simulink model "CCMSysServ"  (R2022b) (continued)
== system CCMSysServ == (continued)
      | TINTED TAILGATE WINDOW
      | <to>All</to>
      | <typ>Internal</typ>
      | <ReqID>NULL</ReqID>
      | <InitValue>0</InitValue>
  CarCfg_CarCfg189 : Inport  Description=|  Port=186  OutDataTypeStr=Nr2  PortDimensions=1
      | REAR SEAT VENTILATION
      | <to>All</to>
      | <typ>Internal</typ>
      | <ReqID>NULL</ReqID>
      | <InitValue>0</InitValue>
  CarCfg_CarCfg19 : Inport  Description=|  Port=187  OutDataTypeStr=Nr2  PortDimensions=1
      | TYRE PRESSURE MONITORING SYSTEM
      | <to>All</to>
      | <typ>Internal</typ>
      | <ReqID>NULL</ReqID>
      | <InitValue>0</InitValue>
  CarCfg_CarCfg190 : Inport  Description=|  Port=188  OutDataTypeStr=Nr2  PortDimensions=1
      | GLOBAL NAVIGATION SAT. SYSTEM RECEIVER
      | <to>All</to>
      | <typ>Internal</typ>
      | <ReqID>NULL</ReqID>
      | <InitValue>0</InitValue>
  CarCfg_CarCfg191 : Inport  Description=|  Port=189  OutDataTypeStr=Nr2  PortDimensions=1
      | TELEMATIC MODULE
      | <to>All</to>
      | <typ>Internal</typ>
      | <ReqID>NULL</ReqID>
      | <InitValue>0</InitValue>
  CarCfg_CarCfg192 : Inport  Description=|  Port=190  OutDataTypeStr=Nr2  PortDimensions=1
      | IHU LEVEL
      | <to>All</to>
      | <typ>Internal</typ>
      | <ReqID>NULL</ReqID>
      | <InitValue>0</InitValue>
  CarCfg_CarCfg193 : Inport  Description=|  Port=191  OutDataTypeStr=Nr2  PortDimensions=1
      | DIGITAL RADIO RECEIVER
      | <to>All</to>
      | <typ>Internal</typ>
      | <ReqID>NULL</ReqID>
      | <InitValue>0</InitValue>
  CarCfg_CarCfg194 : Inport  Description=|  Port=192  OutDataTypeStr=Nr2  PortDimensions=1
      | REAR SEAT ENTERTAINMENT
      | <to>All</to>
      | <typ>Internal</typ>
      | <ReqID>NULL</ReqID>
      | <InitValue>0</InitValue>
  CarCfg_CarCfg195 : Inport  Description=|  Port=193  OutDataTypeStr=Nr2  PortDimensions=1
      | REGION IF DVD
      | <to>All</to>
      | <typ>Internal</typ>
      | <ReqID>NULL</ReqID>
      | <InitValue>0</InitValue>
  CarCfg_CarCfg196 : Inport  Description=|  Port=194  OutDataTypeStr=Nr2  PortDimensions=1
      | Wi-Fi FREQUENCY BAND
      | <to>All</to>
      | <typ>Internal</typ>
      | <ReqID>NULL</ReqID>
      | <InitValue>0</InitValue>
  CarCfg_CarCfg197 : Inport  Description=|  Port=195  OutDataTypeStr=Nr2  PortDimensions=1
      | SYSTEM LANGUAGE
      | <to>All</to>
      | <typ>Internal</typ>
      | <ReqID>NULL</ReqID>
      | <InitValue>0</InitValue>
  CarCfg_CarCfg198 : Inport  Description=|  Port=196  OutDataTypeStr=Nr2  PortDimensions=1
      | SPEECH
      | <to>All</to>
      | <typ>Internal</typ>
      | <ReqID>NULL</ReqID>
      | <InitValue>0</InitValue>
  CarCfg_CarCfg199 : Inport  Description=|  Port=197  OutDataTypeStr=Nr2  PortDimensions=1
      | SPEECH LANGUAGE
      | <to>All</to>
      | <typ>Internal</typ>
      | <ReqID>NULL</ReqID>
      | <InitValue>0</InitValue>
  CarCfg_CarCfg2 : Inport  Description=|  Port=198  OutDataTypeStr=Nr2  PortDimensions=1
      | NUMBER OF DOORS
      | <to>All</to>
      | <typ>Internal</typ>
      | <ReqID>NULL</ReqID>
      | <InitValue>0</InitValue>
  CarCfg_CarCfg20 : Inport  Description=|  Port=199  OutDataTypeStr=Nr2  PortDimensions=1
      | TPMS MENU FUNCTIONS IN IHU
      | <to>All</to>
      | <typ>Internal</typ>
      | <ReqID>NULL</ReqID>
      | <InitValue>0</InitValue>
  CarCfg_CarCfg200 : Inport  Description=|  Port=200  OutDataTypeStr=Nr2  PortDimensions=1
      | Wi-Fi FUNCTIONALITY CONTROL
      | <to>All</to>
      | <typ>Internal</typ>
      | <ReqID>NULL</ReqID>
      | <InitValue>0</InitValue>
  CarCfg_CarCfg201 : Inport  Description=|  Port=201  OutDataTypeStr=Nr2  PortDimensions=1
      | CONNECTIVITY
      | <to>All</to>
      | <typ>Internal</typ>
      | <ReqID>NULL</ReqID>
      | <InitValue>0</InitValue>
  CarCfg_CarCfg202 : Inport  Description=|  Port=202  OutDataTypeStr=Nr2  PortDimensions=1
      | OWNERS MANUAL
      | <to>All</to>
      | <typ>Internal</typ>
      | <ReqID>NULL</ReqID>
      | <InitValue>0</InitValue>
  CarCfg_CarCfg203 : Inport  Description=|  Port=203  OutDataTypeStr=Nr2  PortDimensions=1
      | MESSAGING
      | <to>All</to>
      | <typ>Internal</typ>
      | <ReqID>NULL</ReqID>
      | <InitValue>0</InitValue>
  CarCfg_CarCfg204 : Inport  Description=|  Port=204  OutDataTypeStr=Nr2  PortDimensions=1
      | TV TUNER
      | <to>All</to>
      | <typ>Internal</typ>
      | <ReqID>NULL</ReqID>
      | <InitValue>0</InitValue>
  CarCfg_CarCfg205 : Inport  Description=|  Port=205  OutDataTypeStr=Nr2  PortDimensions=1
      | DSRC UNIT
      | <to>All</to>
      | <typ>Internal</typ>
      | <ReqID>NULL</ReqID>
      | <InitValue>0</InitValue>
  CarCfg_CarCfg206 : Inport  Description=|  Port=206  OutDataTypeStr=Nr2  PortDimensions=1
      | DUAL MEDIA VICS ANTENNA
      | <to>All</to>
      | <typ>Internal</typ>
      | <ReqID>NULL</ReqID>
      | <InitValue>0</InitValue>
  CarCfg_CarCfg207 : Inport  Description=|  Port=207  OutDataTypeStr=Nr2  PortDimensions=1
      | VIDEO ALLOWED
      | <to>All</to>
      | <typ>Internal</typ>
      | <ReqID>NULL</ReqID>
      | <InitValue>0</InitValue>
  CarCfg_CarCfg208 : Inport  Description=|  Port=208  OutDataTypeStr=Nr2  PortDimensions=1
      | DRIVER DISTRACTION PROTECTION
      | <to>All</to>
      | <typ>Internal</typ>
      | <ReqID>NULL</ReqID>
      | <InitValue>0</InitValue>
  CarCfg_CarCfg209 : Inport  Description=|  Port=209  OutDataTypeStr=Nr2  PortDimensions=1
      | ROAD AND TRAFFIC INFO, RTI
      | <to>All</to>
      | <typ>Internal</typ>
      | <ReqID>NULL</ReqID>
      | <InitValue>0</InitValue>
  CarCfg_CarCfg210 : Inport  Description=|  Port=210  OutDataTypeStr=Nr2  PortDimensions=1
      | SUBWOOFER
      | <to>All</to>
      | <typ>Internal</typ>
      | <ReqID>NULL</ReqID>
      | <InitValue>0</InitValue>
  CarCfg_CarCfg211 : Inport  Description=|  Port=211  OutDataTypeStr=Nr2  PortDimensions=1
      | TRAILER MODULE, AFTERMARKET
      | <to>All</to>
      | <typ>Internal</typ>
      | <ReqID>88474</ReqID>
      | <InitValue>0</InitValue>
  CarCfg_CarCfg212 : Inport  Description=|  Port=212  OutDataTypeStr=Nr2  PortDimensions=1
      | ALCOLOCK
      | <to>All</to>
      | <typ>Internal</typ>
      | <ReqID>NULL</ReqID>
      | <InitValue>0</InitValue>
  CarCfg_CarCfg213 : Inport  Description=|  Port=213  OutDataTypeStr=Nr2  PortDimensions=1
      | RUNNINGBOARD, ACCESSORIES
      | <to>All</to>
      | <typ>Internal</typ>
      | <ReqID>NULL</ReqID>
      | <InitValue>0</InitValue>
  CarCfg_CarCfg214 : Inport  Description=|  Port=214  OutDataTypeStr=Nr2  PortDimensions=1
      | RESTRICTED KEY FUNCTION
      | <to>All</to>
      | <typ>Internal</typ>
      | <ReqID>NULL</ReqID>
      | <InitValue>0</InitValue>
  CarCfg_CarCfg215 : Inport  Description=|  Port=215  OutDataTypeStr=Nr2  PortDimensions=1
      | DECO TRIM ILLUMINATION
      | <to>All</to>
      | <typ>Internal</typ>
      | <ReqID>NULL</ReqID>
      | <InitValue>0</InitValue>
  CarCfg_CarCfg216 : Inport  Description=|  Port=216  OutDataTypeStr=Nr2  PortDimensions=1
      | ACCESSORY GATEWAY UNIT
      | <to>All</to>
      | <typ>Internal</typ>
      | <ReqID>NULL</ReqID>
      | <InitValue>0</InitValue>
  CarCfg_CarCfg217 : Inport  Description=|  Port=217  OutDataTypeStr=Nr2  PortDimensions=1
      | EXCELLENCE CHILD SEAT
      | <to>All</to>
      | <typ>Internal</typ>
      | <ReqID>NULL</ReqID>
      | <InitValue>0</InitValue>
  CarCfg_CarCfg218 : Inport  Description=|  Port=218  OutDataTypeStr=Nr2  PortDimensions=1
      | MARKET TELEPHONE NETWORK
      | <to>All</to>
      | <typ>Internal</typ>
      | <ReqID>NULL</ReqID>
      | <InitValue>0</InitValue>
  CarCfg_CarCfg219 : Inport  Description=|  Port=219  OutDataTypeStr=Nr2  PortDimensions=1
      | MICROPHONE SETUP
      | <to>All</to>
      | <typ>Internal</typ>
      | <ReqID>NULL</ReqID>
      | <InitValue>0</InitValue>
  CarCfg_CarCfg22 : Inport  Description=|  Port=220  OutDataTypeStr=Nr2  PortDimensions=1
      | START DEVICE
      | <to>All</to>
      | <typ>Internal</typ>
      | <ReqID>NULL</ReqID>
      | <InitValue>0</InitValue>
  CarCfg_CarCfg220 : Inport  Description=|  Port=221  OutDataTypeStr=Nr2  PortDimensions=1
      | LANGUAGE REGION
      | <to>All</to>
      | <typ>Internal</typ>
      | <ReqID>NULL</ReqID>
      | <InitValue>0</InitValue>
  CarCfg_CarCfg221 : Inport  Description=|  Port=222  OutDataTypeStr=Nr2  PortDimensions=1
      | CONNECTED SERVICE BOOKING, IF CONNECTIVITY
      | <to>All</to>
      | <typ>Internal</typ>
      | <ReqID>NULL</ReqID>
      | <InitValue>0</InitValue>
  CarCfg_CarCfg222 : Inport  Description=|  Port=223  OutDataTypeStr=Nr2  PortDimensions=1
      | MEDIAPLAYER
      | <to>All</to>
      | <typ>Internal</typ>
      | <ReqID>NULL</ReqID>
      | <InitValue>0</InitValue>
  CarCfg_CarCfg223 : Inport  Description=|  Port=224  OutDataTypeStr=Nr2  PortDimensions=1
      | NAVIGATION SYSTEM AND INTERNET MAP
      | <to>All</to>
      | <typ>Internal</typ>
      | <ReqID>NULL</ReqID>
      | <InitValue>0</InitValue>
  CarCfg_CarCfg224 : Inport  Description=|  Port=225  OutDataTypeStr=Nr2  PortDimensions=1
      | DEVICE MIRRORING
      | <to>All</to>
      | <typ>Internal</typ>
      | <ReqID>NULL</ReqID>
      | <InitValue>0</InitValue>
  CarCfg_CarCfg225 : Inport  Description=|  Port=226  OutDataTypeStr=Nr2  PortDimensions=1
      | TYRE PRESSURE LLF
      | <to>All</to>
      | <typ>Internal</typ>
      | <ReqID>NULL</ReqID>
      | <InitValue>0</InitValue>
  CarCfg_CarCfg226 : Inport  Description=|  Port=227  OutDataTypeStr=Nr2  PortDimensions=1
      | TYRE PRESSURE LLR
      | <to>All</to>
      | <typ>Internal</typ>
      | <ReqID>NULL</ReqID>
      | <InitValue>0</InitValue>
  CarCfg_CarCfg227 : Inport  Description=|  Port=228  OutDataTypeStr=Nr2  PortDimensions=1
      | TYRE PRESSURE LHF
      | <to>All</to>
      | <typ>Internal</typ>
      | <ReqID>NULL</ReqID>
      | <InitValue>0</InitValue>
  CarCfg_CarCfg228 : Inport  Description=|  Port=229  OutDataTypeStr=Nr2  PortDimensions=1
      | TYRE PRESSURE LHR
      | <to>All</to>
      | <typ>Internal</typ>
      | <ReqID>NULL</ReqID>
      | <InitValue>0</InitValue>
  CarCfg_CarCfg229 : Inport  Description=|  Port=230  OutDataTypeStr=Nr2  PortDimensions=1
      | TYRE PRESSURE HLF
      | <to>All</to>
      | <typ>Internal</typ>
      | <ReqID>NULL</ReqID>
      | <InitValue>0</InitValue>
  CarCfg_CarCfg23 : Inport  Description=|  Port=231  OutDataTypeStr=Nr2  PortDimensions=1
      | CRUISE CONTROL
      | <to>All</to>
      | <typ>Internal</typ>
      | <ReqID>NULL</ReqID>
      | <InitValue>0</InitValue>
  CarCfg_CarCfg230 : Inport  Description=|  Port=232  OutDataTypeStr=Nr2  PortDimensions=1
      | TYRE PRESSURE HLR
      | <to>All</to>
      | <typ>Internal</typ>
      | <ReqID>NULL</ReqID>
      | <InitValue>0</InitValue>
  CarCfg_CarCfg231 : Inport  Description=|  Port=233  OutDataTypeStr=Nr2  PortDimensions=1
      | TYRE PRESSURE HHF
      | <to>All</to>
      | <typ>Internal</typ>
      | <ReqID>NULL</ReqID>
      | <InitValue>0</InitValue>
  CarCfg_CarCfg232 : Inport  Description=|  Port=234  OutDataTypeStr=Nr2  PortDimensions=1
      | TYRE PRESSURE HHR
      | <to>All</to>
      | <typ>Internal</typ>
      | <ReqID>NULL</ReqID>
      | <InitValue>0</InitValue>
  CarCfg_CarCfg235 : Inport  Description=|  Port=235  OutDataTypeStr=Nr2  PortDimensions=1
      | PHONE AS KEY
      | <to>All</to>
      | <typ>Internal</typ>
      | <ReqID>NULL</ReqID>
      | <InitValue>0</InitValue>
  CarCfg_CarCfg236 : Inport  Description=|  Port=236  OutDataTypeStr=Nr2  PortDimensions=1
      | EXTENDED ACCESSORY MODULE FUNCTIONALLITY
      | <to>All</to>
      | <typ>Internal</typ>
      | <ReqID>NULL</ReqID>
      | <InitValue>0</InitValue>
  CarCfg_CarCfg237 : Inport  Description=|  Port=237  OutDataTypeStr=Nr2  PortDimensions=1
      | DEFAULT RIDE HEIGHT
      | <to>All</to>
      | <typ>Internal</typ>
      | <ReqID>NULL</ReqID>
      | <InitValue>0</InitValue>
  CarCfg_CarCfg238 : Inport  Description=|  Port=238  OutDataTypeStr=Nr2  PortDimensions=1
      | TOP DAMPING, BRAKES
      | <to>All</to>
      | <typ>Internal</typ>
      | <ReqID>NULL</ReqID>
      | <InitValue>0</InitValue>
  CarCfg_CarCfg239 : Inport  Description=|  Port=239  OutDataTypeStr=Nr2  PortDimensions=1
      | MASSAGE FRONT SEAT
      | <to>All</to>
      | <typ>Internal</typ>
      | <ReqID>NULL</ReqID>
      | <InitValue>0</InitValue>
  CarCfg_CarCfg24 : Inport  Description=|  Port=240  OutDataTypeStr=Nr2  PortDimensions=1
      | SEATS
      | <to>All</to>
      | <typ>Internal</typ>
      | <ReqID>NULL</ReqID>
      | <InitValue>0</InitValue>
  CarCfg_CarCfg240 : Inport  Description=|  Port=241  OutDataTypeStr=Nr2  PortDimensions=1
      | LUMBAR SUPPORT
      | <to>All</to>
      | <typ>Internal</typ>
      | <ReqID>NULL</ReqID>
      | <InitValue>0</InitValue>
  CarCfg_CarCfg241 : Inport  Description=|  Port=242  OutDataTypeStr=Nr2  PortDimensions=1
      | CUSHION EXTENSION
      | <to>All</to>
      | <typ>Internal</typ>
      | <ReqID>NULL</ReqID>
      | <InitValue>0</InitValue>
  CarCfg_CarCfg242 : Inport  Description=|  Port=243  OutDataTypeStr=Nr2  PortDimensions=1
      | HEADRESTS FRONT
      | <to>All</to>
      | <typ>Internal</typ>
      | <ReqID>NULL</ReqID>
      | <InitValue>0</InitValue>
  CarCfg_CarCfg243 : Inport  Description=|  Port=244  OutDataTypeStr=Nr2  PortDimensions=1
      | POWER ADJUSTABLE SIDE SUPPORT
      | <to>All</to>
      | <typ>Internal</typ>
      | <ReqID>NULL</ReqID>
      | <InitValue>0</InitValue>
  CarCfg_CarCfg244 : Inport  Description=|  Port=245  OutDataTypeStr=Nr2  PortDimensions=1
      | REMOTE CONTROL OF PASSENGER SEAT FUNCTIONS
      | <to>All</to>
      | <typ>Internal</typ>
      | <ReqID>NULL</ReqID>
      | <InitValue>0</InitValue>
  CarCfg_CarCfg245 : Inport  Description=|  Port=246  OutDataTypeStr=Nr2  PortDimensions=1
      | EASY INGRESS EGRESS
      | <to>All</to>
      | <typ>Internal</typ>
      | <ReqID>NULL</ReqID>
      | <InitValue>0</InitValue>
  CarCfg_CarCfg246 : Inport  Description=|  Port=247  OutDataTypeStr=Nr2  PortDimensions=1
      | POWER RELEASE 2:ND ROW
      | <to>All</to>
      | <typ>Internal</typ>
      | <ReqID>NULL</ReqID>
      | <InitValue>0</InitValue>
  CarCfg_CarCfg247 : Inport  Description=|  Port=248  OutDataTypeStr=Nr2  PortDimensions=1
      | HVAC FILTER TYPE
      | <to>All</to>
      | <typ>Internal</typ>
      | <ReqID>NULL</ReqID>
      | <InitValue>0</InitValue>
  CarCfg_CarCfg248 : Inport  Description=|  Port=249  OutDataTypeStr=Nr2  PortDimensions=1
      | IAQS SMOKE SENSOR
      | <to>All</to>
      | <typ>Internal</typ>
      | <ReqID>NULL</ReqID>
      | <InitValue>0</InitValue>
  CarCfg_CarCfg249 : Inport  Description=|  Port=250  OutDataTypeStr=Nr2  PortDimensions=1
      | OVERHEAD CONSOLE TYPE
      | <to>All</to>
      | <typ>Internal</typ>
      | <ReqID>NULL</ReqID>
      | <InitValue>0</InitValue>
  CarCfg_CarCfg25 : Inport  Description=|  Port=251  OutDataTypeStr=Nr2  PortDimensions=1
      | AVP TYPE
      | <to>All</to>
      | <typ>Internal</typ>
      | <ReqID>NULL</ReqID>
      | <InitValue>0</InitValue>
  CarCfg_CarCfg250 : Inport  Description=|  Port=252  OutDataTypeStr=Nr2  PortDimensions=1
      | ELECTRIC DISTANCE TO EMPTY
      | <to>All</to>
      | <typ>Internal</typ>
      | <ReqID>NULL</ReqID>
      | <InitValue>0</InitValue>
  CarCfg_CarCfg253 : Inport  Description=|  Port=253  OutDataTypeStr=Nr2  PortDimensions=1
      | FRONT DIRECTION INDICATOR LAMP TYPE
      | <to>All</to>
      | <typ>Internal</typ>
      | <ReqID>NULL</ReqID>
      | <InitValue>0</InitValue>
  CarCfg_CarCfg254 : Inport  Description=|  Port=254  OutDataTypeStr=Nr2  PortDimensions=1
      | REAR DIRECTION INDICATOR LAMP TYPE
      | <to>All</to>
      | <typ>Internal</typ>
      | <ReqID>NULL</ReqID>
      | <InitValue>0</InitValue>
  CarCfg_CarCfg255 : Inport  Description=|  Port=255  OutDataTypeStr=Nr2  PortDimensions=1
      | REAR FOGLIGHT LAMP TYPE
      | <to>All</to>
      | <typ>Internal</typ>
      | <ReqID>NULL</ReqID>
      | <InitValue>0</InitValue>
  CarCfg_CarCfg256 : Inport  Description=|  Port=256  OutDataTypeStr=Nr2  PortDimensions=1
      | BENDING LIGHT
      | <to>All</to>
      | <typ>Internal</typ>
      | <ReqID>NULL</ReqID>
      | <InitValue>0</InitValue>
  CarCfg_CarCfg257 : Inport  Description=|  Port=257  OutDataTypeStr=Nr2  PortDimensions=1
      | REAR POSITION LAMP TYPE
      | <to>All</to>
      | <typ>Internal</typ>
      | <ReqID>NULL</ReqID>
      | <InitValue>0</InitValue>
  CarCfg_CarCfg258 : Inport  Description=|  Port=258  OutDataTypeStr=Nr2  PortDimensions=1
      | REAR STOP LEFT/RIGHT LAMP TYPE
      | <to>All</to>
      | <typ>Internal</typ>
      | <ReqID>NULL</ReqID>
      | <InitValue>0</InitValue>
  CarCfg_CarCfg259 : Inport  Description=|  Port=259  OutDataTypeStr=Nr2  PortDimensions=1
      | REVERSING LAMP TYPE
      | <to>All</to>
      | <typ>Internal</typ>
      | <ReqID>NULL</ReqID>
      | <InitValue>0</InitValue>
  CarCfg_CarCfg262 : Inport  Description=|  Port=260  OutDataTypeStr=Nr2  PortDimensions=1
      | THERMAL MANAGEMENT
      | <to>All</to>
      | <typ>Internal</typ>
      | <ReqID>NULL</ReqID>
      | <InitValue>0</InitValue>
  CarCfg_CarCfg263 : Inport  Description=|  Port=261  OutDataTypeStr=Nr2  PortDimensions=1
      | HEAT PUMP
      | <to>All</to>
      | <typ>Internal</typ>
      | <ReqID>NULL</ReqID>
      | <InitValue>0</InitValue>
  CarCfg_CarCfg267 : Inport  Description=|  Port=262  OutDataTypeStr=Nr2  PortDimensions=1
      | INDIRECT TPMS SYSTEM
      | <to>All</to>
      | <typ>Internal</typ>
      | <ReqID>NULL</ReqID>
      | <InitValue>0</InitValue>
  CarCfg_CarCfg268 : Inport  Description=|  Port=263  OutDataTypeStr=Nr2  PortDimensions=1
      | NUMBER OF CYLINDERS
      | <to>All</to>
      | <typ>Internal</typ>
      | <ReqID>NULL</ReqID>
      | <InitValue>0</InitValue>
  CarCfg_CarCfg270 : Inport  Description=|  Port=264  OutDataTypeStr=Nr2  PortDimensions=1
      | VEM INFOTAINMENT SETTINGS
      | <to>All</to>
      | <typ>Internal</typ>
      | <ReqID>NULL</ReqID>
      | <InitValue>0</InitValue>
  CarCfg_CarCfg271 : Inport  Description=|  Port=265  OutDataTypeStr=Nr2  PortDimensions=1
      | STOP/START HMI SETTINGS
      | <to>All</to>
      | <typ>Internal</typ>
      | <ReqID>NULL</ReqID>
      | <InitValue>0</InitValue>
  CarCfg_CarCfg273 : Inport  Description=|  Port=266  OutDataTypeStr=Nr2  PortDimensions=1
      | ADAPTIVE REAR LIGHT (ARL) AVAILABILITY
      | <to>All</to>
      | <typ>Internal</typ>
      | <ReqID>NULL</ReqID>
      | <InitValue>0</InitValue>
  CarCfg_CarCfg274 : Inport  Description=|  Port=267  OutDataTypeStr=Nr2  PortDimensions=1
      | HEADLAMP PROJECT, FOR HCM
      | <to>All</to>
      | <typ>Internal</typ>
      | <ReqID>NULL</ReqID>
      | <InitValue>0</InitValue>
  CarCfg_CarCfg275 : Inport  Description=|  Port=268  OutDataTypeStr=Nr2  PortDimensions=1
      | REAR SEAT REMOTE FUNCTIONALITY
      | <to>All</to>
      | <typ>Internal</typ>
      | <ReqID>NULL</ReqID>
      | <InitValue>0</InitValue>
  CarCfg_CarCfg28 : Inport  Description=|  Port=269  OutDataTypeStr=Nr2  PortDimensions=1
      | RADIO, AMP & SPEAKER SYSTEM
      | <to>All</to>
      | <typ>Internal</typ>
      | <ReqID>NULL</ReqID>
      | <InitValue>0</InitValue>
  CarCfg_CarCfg289 : Inport  Description=|  Port=270  OutDataTypeStr=Nr2  PortDimensions=1
      | ESCALATED EMERGENCY STOP
      | <to>All</to>
      | <typ>Internal</typ>
      | <ReqID>NULL</ReqID>
      | <InitValue>0</InitValue>
  CarCfg_CarCfg290 : Inport  Description=|  Port=271  OutDataTypeStr=Nr2  PortDimensions=1
      | OCCUPANT SAFE POSITIONING
      | <to>All</to>
      | <typ>Internal</typ>
      | <ReqID>NULL</ReqID>
      | <InitValue>0</InitValue>
  CarCfg_CarCfg3 : Inport  Description=|  Port=272  OutDataTypeStr=Nr2  PortDimensions=1
      | TRANSMISSION DRIVELINE
      | <to>All</to>
      | <typ>Internal</typ>
      | <ReqID>NULL</ReqID>
      | <InitValue>0</InitValue>
  CarCfg_CarCfg30 : Inport  Description=|  Port=273  OutDataTypeStr=Nr2  PortDimensions=1
      | BATTERY
      | <to>All</to>
      | <typ>Internal</typ>
      | <ReqID>NULL</ReqID>
      | <InitValue>0</InitValue>
  CarCfg_CarCfg301 : Inport  Description=|  Port=274  OutDataTypeStr=Nr2  PortDimensions=1
      | DRIVER AIRBAG TYPE
      | <to>All</to>
      | <typ>Internal</typ>
      | <ReqID>NULL</ReqID>
      | <InitValue>0</InitValue>
  CarCfg_CarCfg302 : Inport  Description=|  Port=275  OutDataTypeStr=Nr2  PortDimensions=1
      | RSDS VERSION
      | <to>All</to>
      | <typ>Internal</typ>
      | <ReqID>NULL</ReqID>
      | <InitValue>0</InitValue>
  CarCfg_CarCfg303 : Inport  Description=|  Port=276  OutDataTypeStr=Nr2  PortDimensions=1
      | REAR SUBFRAME FASTENING
      | <to>All</to>
      | <typ>Internal</typ>
      | <ReqID>NULL</ReqID>
      | <InitValue>0</InitValue>
  CarCfg_CarCfg304 : Inport  Description=|  Port=277  OutDataTypeStr=Nr2  PortDimensions=1
      | KNEE AIRBAG, 2-STAGES
      | <to>All</to>
      | <typ>Internal</typ>
      | <ReqID>NULL</ReqID>
      | <InitValue>0</InitValue>
  CarCfg_CarCfg305 : Inport  Description=|  Port=278  OutDataTypeStr=Nr2  PortDimensions=1
      | PARKING ASSISTANCE SENSOR GENERATION
      | <to>All</to>
      | <typ>Internal</typ>
      | <ReqID>NULL</ReqID>
      | <InitValue>0</InitValue>
  CarCfg_CarCfg307 : Inport  Description=|  Port=279  OutDataTypeStr=Nr2  PortDimensions=1
      | C-PILLAR SENSOR
      | <to>All</to>
      | <typ>Internal</typ>
      | <ReqID>NULL</ReqID>
      | <InitValue>0</InitValue>
  CarCfg_CarCfg308 : Inport  Description=|  Port=280  OutDataTypeStr=Nr2  PortDimensions=1
      | SRS MARKET VARIANT
      | <to>All</to>
      | <typ>Internal</typ>
      | <ReqID>NULL</ReqID>
      | <InitValue>0</InitValue>
  CarCfg_CarCfg309 : Inport  Description=|  Port=281  OutDataTypeStr=Nr2  PortDimensions=1
      | FORWARD LOOKING RADAR
      | <to>All</to>
      | <typ>Internal</typ>
      | <ReqID>NULL</ReqID>
      | <InitValue>0</InitValue>
  CarCfg_CarCfg310 : Inport  Description=|  Port=282  OutDataTypeStr=Nr2  PortDimensions=1
      | FRONT SIDE RADARS
      | <to>All</to>
      | <typ>Internal</typ>
      | <ReqID>NULL</ReqID>
      | <InitValue>0</InitValue>
  CarCfg_CarCfg311 : Inport  Description=|  Port=283  OutDataTypeStr=Nr2  PortDimensions=1
      | FLC VARIANT
      | <to>All</to>
      | <typ>Internal</typ>
      | <ReqID>NULL</ReqID>
      | <InitValue>0</InitValue>
  CarCfg_CarCfg312 : Inport  Description=|  Port=284  OutDataTypeStr=Nr2  PortDimensions=1
      | LANE CHANGE ASSIST
      | <to>All</to>
      | <typ>Internal</typ>
      | <ReqID>NULL</ReqID>
      | <InitValue>0</InitValue>
  CarCfg_CarCfg313 : Inport  Description=|  Port=285  OutDataTypeStr=Nr2  PortDimensions=1
      | SAFETY COACH
      | <to>All</to>
      | <typ>Internal</typ>
      | <ReqID>NULL</ReqID>
      | <InitValue>0</InitValue>
  CarCfg_CarCfg314 : Inport  Description=|  Port=286  OutDataTypeStr=Nr2  PortDimensions=1
      | ADAPTIVE CRUISE CONTROL VERSION
      | <to>All</to>
      | <typ>Internal</typ>
      | <ReqID>NULL</ReqID>
      | <InitValue>0</InitValue>
  CarCfg_CarCfg315 : Inport  Description=|  Port=287  OutDataTypeStr=Nr2  PortDimensions=1
      | READY TO DRIVE NOTIFICATION
      | <to>All</to>
      | <typ>Internal</typ>
      | <ReqID>NULL</ReqID>
      | <InitValue>0</InitValue>
  CarCfg_CarCfg316 : Inport  Description=|  Port=288  OutDataTypeStr=Nr2  PortDimensions=1
      | EMERGENCY LANE KEEPING AID
      | <to>All</to>
      | <typ>Internal</typ>
      | <ReqID>NULL</ReqID>
      | <InitValue>0</InitValue>
  CarCfg_CarCfg317 : Inport  Description=|  Port=289  OutDataTypeStr=Nr2  PortDimensions=1
      | EVASIVE MANOUEVRE ASSIST
      | <to>All</to>
      | <typ>Internal</typ>
      | <ReqID>NULL</ReqID>
      | <InitValue>0</InitValue>
  CarCfg_CarCfg318 : Inport  Description=|  Port=290  OutDataTypeStr=Nr2  PortDimensions=1
      | AUTO PILOT FUNCTION (HAD )
      | <to>All</to>
      | <typ>Internal</typ>
      | <ReqID>NULL</ReqID>
      | <InitValue>0</InitValue>
  CarCfg_CarCfg319 : Inport  Description=|  Port=291  OutDataTypeStr=Nr2  PortDimensions=1
      | SAFETY BELT REMINDER 2ND ROW
      | <to>All</to>
      | <typ>Internal</typ>
      | <ReqID>NULL</ReqID>
      | <InitValue>0</InitValue>
  CarCfg_CarCfg32 : Inport  Description=|  Port=292  OutDataTypeStr=Nr2  PortDimensions=1
      | LOW VOLTAGE ELECTRICAL CONFIGURATION
      | <to>All</to>
      | <typ>Internal</typ>
      | <ReqID>NULL</ReqID>
      | <InitValue>0</InitValue>
  CarCfg_CarCfg321 : Inport  Description=|  Port=293  OutDataTypeStr=Nr2  PortDimensions=1
      | BRAKE PULSE INTERFACE
      | <to>All</to>
      | <typ>Internal</typ>
      | <ReqID>NULL</ReqID>
      | <InitValue>0</InitValue>
  CarCfg_CarCfg322 : Inport  Description=|  Port=294  OutDataTypeStr=Nr2  PortDimensions=1
      | CMS ONCOMING
      | <to>All</to>
      | <typ>Internal</typ>
      | <ReqID>NULL</ReqID>
      | <InitValue>0</InitValue>
  CarCfg_CarCfg323 : Inport  Description=|  Port=295  OutDataTypeStr=Nr2  PortDimensions=1
      | REAR AUTOMATIC EMERGENCY BRAKING
      | <to>All</to>
      | <typ>Internal</typ>
      | <ReqID>NULL</ReqID>
      | <InitValue>0</InitValue>
  CarCfg_CarCfg324 : Inport  Description=|  Port=296  OutDataTypeStr=Nr2  PortDimensions=1
      | VACUUM LESS BRAKE SYSTEM
      | <to>All</to>
      | <typ>Internal</typ>
      | <ReqID>NULL</ReqID>
      | <InitValue>0</InitValue>
  CarCfg_CarCfg325 : Inport  Description=|  Port=297  OutDataTypeStr=Nr2  PortDimensions=1
      | PEDESTRIAN PROTECTION SYSTEM SENSOR CONFIGURATION
      | <to>All</to>
      | <typ>Internal</typ>
      | <ReqID>NULL</ReqID>
      | <InitValue>0</InitValue>
  CarCfg_CarCfg33 : Inport  Description=|  Port=298  OutDataTypeStr=Nr2  PortDimensions=1
      | STRAW IN FUEL RESERVOIR
      | <to>All</to>
      | <typ>Internal</typ>
      | <ReqID>NULL</ReqID>
      | <InitValue>0</InitValue>
  CarCfg_CarCfg335 : Inport  Description=|  Port=299  OutDataTypeStr=Nr2  PortDimensions=1
      | HVAC FLOOR SENSORS
      | <to>All</to>
      | <typ>Internal</typ>
      | <ReqID>NULL</ReqID>
      | <InitValue>0</InitValue>
  CarCfg_CarCfg336 : Inport  Description=|  Port=300  OutDataTypeStr=Nr2  PortDimensions=1
      | AIRBORNE PARTICULATE MATTER SENSOR
      | <to>All</to>
      | <typ>Internal</typ>
      | <ReqID>NULL</ReqID>
      | <InitValue>0</InitValue>
  CarCfg_CarCfg337 : Inport  Description=|  Port=301  OutDataTypeStr=Nr2  PortDimensions=1
      | CENTER CONSOLE SWITCH MODULE(CCSM)
      | <to>All</to>
      | <typ>Internal</typ>
      | <ReqID>NULL</ReqID>
      | <InitValue>0</InitValue>
  CarCfg_CarCfg338 : Inport  Description=|  Port=302  OutDataTypeStr=Nr2  PortDimensions=1
      | PRE_CLEAN
      | <to>All</to>
      | <typ>Internal</typ>
      | <ReqID>NULL</ReqID>
      | <InitValue>0</InitValue>
  CarCfg_CarCfg339 : Inport  Description=|  Port=303  OutDataTypeStr=Nr2  PortDimensions=1
      | SECOND ROW CENTRE VENT
      | <to>All</to>
      | <typ>Internal</typ>
      | <ReqID>NULL</ReqID>
      | <InitValue>0</InitValue>
  CarCfg_CarCfg34 : Inport  Description=|  Port=304  OutDataTypeStr=Nr2  PortDimensions=1
      | ENGINE THERMOSTAT HEATING
      | <to>All</to>
      | <typ>Internal</typ>
      | <ReqID>NULL</ReqID>
      | <InitValue>0</InitValue>
  CarCfg_CarCfg340 : Inport  Description=|  Port=305  OutDataTypeStr=Nr2  PortDimensions=1
      | ADVANCE AIR CLEANER IONIZER
      | <to>All</to>
      | <typ>Internal</typ>
      | <ReqID>NULL</ReqID>
      | <InitValue>0</InitValue>
  CarCfg_CarCfg341 : Inport  Description=|  Port=306  OutDataTypeStr=Nr2  PortDimensions=1
      | CLIMATE SMALL COOLANT CIRCUIT
      | <to>All</to>
      | <typ>Internal</typ>
      | <ReqID>NULL</ReqID>
      | <InitValue>0</InitValue>
  CarCfg_CarCfg342 : Inport  Description=|  Port=307  OutDataTypeStr=Nr2  PortDimensions=1
      | CLIMATE 2-WAY COOLANT VALVE
      | <to>All</to>
      | <typ>Internal</typ>
      | <ReqID>NULL</ReqID>
      | <InitValue>0</InitValue>
  CarCfg_CarCfg343 : Inport  Description=|  Port=308  OutDataTypeStr=Nr2  PortDimensions=1
      | CLIMATE AUX WATER PUMP
      | <to>All</to>
      | <typ>Internal</typ>
      | <ReqID>NULL</ReqID>
      | <InitValue>0</InitValue>
  CarCfg_CarCfg344 : Inport  Description=|  Port=309  OutDataTypeStr=Nr2  PortDimensions=1
      | LAMINATED WINDOWS
      | <to>All</to>
      | <typ>Internal</typ>
      | <ReqID>NULL</ReqID>
      | <InitValue>0</InitValue>
  CarCfg_CarCfg345 : Inport  Description=|  Port=310  OutDataTypeStr=Nr2  PortDimensions=1
      | BYPASS VALVE HVAC
      | <to>All</to>
      | <typ>Internal</typ>
      | <ReqID>NULL</ReqID>
      | <InitValue>0</InitValue>
  CarCfg_CarCfg346 : Inport  Description=|  Port=311  OutDataTypeStr=Nr2  PortDimensions=1
      | SUN CURTAIN REAR WINDOW
      | <to>All</to>
      | <typ>Internal</typ>
      | <ReqID>NULL</ReqID>
      | <InitValue>0</InitValue>
  CarCfg_CarCfg347 : Inport  Description=|  Port=312  OutDataTypeStr=Nr2  PortDimensions=1
      | HEATER TYPE
      | <to>All</to>
      | <typ>Internal</typ>
      | <ReqID>NULL</ReqID>
      | <InitValue>0</InitValue>
  CarCfg_CarCfg348 : Inport  Description=|  Port=313  OutDataTypeStr=Nr2  PortDimensions=1
      | HEX-TEMPERATURE SENSOR
      | <to>All</to>
      | <typ>Internal</typ>
      | <ReqID>NULL</ReqID>
      | <InitValue>0</InitValue>
  CarCfg_CarCfg349 : Inport  Description=|  Port=314  OutDataTypeStr=Nr2  PortDimensions=1
      | IONIC INTERNAL AIR CLEANER
      | <to>All</to>
      | <typ>Internal</typ>
      | <ReqID>NULL</ReqID>
      | <InitValue>0</InitValue>
  CarCfg_CarCfg35 : Inport  Description=|  Port=315  OutDataTypeStr=Nr2  PortDimensions=1
      | OIL SUMP TYPE
      | <to>All</to>
      | <typ>Internal</typ>
      | <ReqID>NULL</ReqID>
      | <InitValue>0</InitValue>
  CarCfg_CarCfg350 : Inport  Description=|  Port=316  OutDataTypeStr=Nr2  PortDimensions=1
      | MEMORY BUTTONS, IF POWER SEAT
      | <to>All</to>
      | <typ>Internal</typ>
      | <ReqID>NULL</ReqID>
      | <InitValue>0</InitValue>
  CarCfg_CarCfg351 : Inport  Description=|  Port=317  OutDataTypeStr=Nr2  PortDimensions=1
      | SUN CURTAIN SIDEWINDOW REAR
      | <to>All</to>
      | <typ>Internal</typ>
      | <ReqID>NULL</ReqID>
      | <InitValue>0</InitValue>
  CarCfg_CarCfg352 : Inport  Description=|  Port=318  OutDataTypeStr=Nr2  PortDimensions=1
      | SUN SENSOR
      | <to>All</to>
      | <typ>Internal</typ>
      | <ReqID>NULL</ReqID>
      | <InitValue>0</InitValue>
  CarCfg_CarCfg353 : Inport  Description=|  Port=319  OutDataTypeStr=Nr2  PortDimensions=1
      | HUMIDITY SENSOR
      | <to>All</to>
      | <typ>Internal</typ>
      | <ReqID>NULL</ReqID>
      | <InitValue>0</InitValue>
  CarCfg_CarCfg354 : Inport  Description=|  Port=320  OutDataTypeStr=Nr2  PortDimensions=1
      | COMPARTMENT TEMPERATURE SENSOR
      | <to>All</to>
      | <typ>Internal</typ>
      | <ReqID>NULL</ReqID>
      | <InitValue>0</InitValue>
  CarCfg_CarCfg355 : Inport  Description=|  Port=321  OutDataTypeStr=Nr2  PortDimensions=1
      | PTC IN FRONT HVAC
      | <to>All</to>
      | <typ>Internal</typ>
      | <ReqID>NULL</ReqID>
      | <InitValue>0</InitValue>
  CarCfg_CarCfg356 : Inport  Description=|  Port=322  OutDataTypeStr=Nr2  PortDimensions=1
      | SECOND ROW SIDE VENT
      | <to>All</to>
      | <typ>Internal</typ>
      | <ReqID>NULL</ReqID>
      | <InitValue>0</InitValue>
  CarCfg_CarCfg357 : Inport  Description=|  Port=323  OutDataTypeStr=Nr2  PortDimensions=1
      | REAR PASSENGER DOOR SWITCHPACK
      | <to>All</to>
      | <typ>Internal</typ>
      | <ReqID>NULL</ReqID>
      | <InitValue>0</InitValue>
  CarCfg_CarCfg358 : Inport  Description=|  Port=324  OutDataTypeStr=Nr2  PortDimensions=1
      | ACTIVE NOISE CONTROL
      | <to>All</to>
      | <typ>Internal</typ>
      | <ReqID>NULL</ReqID>
      | <InitValue>0</InitValue>
  CarCfg_CarCfg359 : Inport  Description=|  Port=325  OutDataTypeStr=Nr2  PortDimensions=1
      | EXTERIOR SOUND HMI OPTION
      | <to>All</to>
      | <typ>Internal</typ>
      | <ReqID>NULL</ReqID>
      | <InitValue>0</InitValue>
  CarCfg_CarCfg36 : Inport  Description=|  Port=326  OutDataTypeStr=Nr2  PortDimensions=1
      | ADJUSTABLE SPEED LIMITER
      | <to>All</to>
      | <typ>Internal</typ>
      | <ReqID>NULL</ReqID>
      | <InitValue>0</InitValue>
  CarCfg_CarCfg360 : Inport  Description=|  Port=327  OutDataTypeStr=Nr2  PortDimensions=1
      | FREQUENCY BAND & STEP, AM/FM HD RADIO
      | <to>All</to>
      | <typ>Internal</typ>
      | <ReqID>NULL</ReqID>
      | <InitValue>0</InitValue>
  CarCfg_CarCfg361 : Inport  Description=|  Port=328  OutDataTypeStr=Nr2  PortDimensions=1
      | SIM CARD FOR CONNECTIVITY
      | <to>All</to>
      | <typ>Internal</typ>
      | <ReqID>NULL</ReqID>
      | <InitValue>0</InitValue>
  CarCfg_CarCfg362 : Inport  Description=|  Port=329  OutDataTypeStr=Nr2  PortDimensions=1
      | USB CONNECTION
      | <to>All</to>
      | <typ>Internal</typ>
      | <ReqID>NULL</ReqID>
      | <InitValue>0</InitValue>
  CarCfg_CarCfg363 : Inport  Description=|  Port=330  OutDataTypeStr=Nr2  PortDimensions=1
      | AUDIO AUXILIARY INPUT
      | <to>All</to>
      | <typ>Internal</typ>
      | <ReqID>NULL</ReqID>
      | <InitValue>0</InitValue>
  CarCfg_CarCfg364 : Inport  Description=|  Port=331  OutDataTypeStr=Nr2  PortDimensions=1
      | EXTERIOR SOUND MODULE CALIBRATION TYPES
      | <to>All</to>
      | <typ>Internal</typ>
      | <ReqID>NULL</ReqID>
      | <InitValue>0</InitValue>
  CarCfg_CarCfg365 : Inport  Description=|  Port=332  OutDataTypeStr=Nr2  PortDimensions=1
      | Wi-Fi WORKSHOP MODE FUNCTIONAL CONTROL
      | <to>All</to>
      | <typ>Internal</typ>
      | <ReqID>NULL</ReqID>
      | <InitValue>0</InitValue>
  CarCfg_CarCfg366 : Inport  Description=|  Port=333  OutDataTypeStr=Nr2  PortDimensions=1
      | WIRELESS PHONE CHARGER
      | <to>All</to>
      | <typ>Internal</typ>
      | <ReqID>NULL</ReqID>
      | <InitValue>0</InitValue>
  CarCfg_CarCfg367 : Inport  Description=|  Port=334  OutDataTypeStr=Nr2  PortDimensions=1
      | REMOTE BATTERY MANAGEMENT
      | <to>All</to>
      | <typ>Internal</typ>
      | <ReqID>NULL</ReqID>
      | <InitValue>0</InitValue>
  CarCfg_CarCfg368 : Inport  Description=|  Port=335  OutDataTypeStr=Nr2  PortDimensions=1
      | ENGINE FAMILY TYPE
      | <to>All</to>
      | <typ>Internal</typ>
      | <ReqID>NULL</ReqID>
      | <InitValue>0</InitValue>
  CarCfg_CarCfg369 : Inport  Description=|  Port=336  OutDataTypeStr=Nr2  PortDimensions=1
      | HV BATTERY SYMBOL
      | <to>All</to>
      | <typ>Internal</typ>
      | <ReqID>NULL</ReqID>
      | <InitValue>0</InitValue>
  CarCfg_CarCfg37 : Inport  Description=|  Port=337  OutDataTypeStr=Nr2  PortDimensions=1
      | GEARSHIFT INDICATOR
      | <to>All</to>
      | <typ>Internal</typ>
      | <ReqID>NULL</ReqID>
      | <InitValue>0</InitValue>
  CarCfg_CarCfg371 : Inport  Description=|  Port=338  OutDataTypeStr=Nr2  PortDimensions=1
      | EXTERIOR SOUND SYSTEM
      | <to>All</to>
      | <typ>Internal</typ>
      | <ReqID>NULL</ReqID>
      | <InitValue>0</InitValue>
  CarCfg_CarCfg372 : Inport  Description=|  Port=339  OutDataTypeStr=Nr2  PortDimensions=1
      | TELEMATICS NODE TYPE
      | <to>All</to>
      | <typ>Internal</typ>
      | <ReqID>NULL</ReqID>
      | <InitValue>0</InitValue>
  CarCfg_CarCfg373 : Inport  Description=|  Port=340  OutDataTypeStr=Nr2  PortDimensions=1
      | PREMIUM TMC
      | <to>All</to>
      | <typ>Internal</typ>
      | <ReqID>NULL</ReqID>
      | <InitValue>0</InitValue>
  CarCfg_CarCfg374 : Inport  Description=|  Port=341  OutDataTypeStr=Nr2  PortDimensions=1
      | POLESTAR LEVEL
      | <to>All</to>
      | <typ>Internal</typ>
      | <ReqID>NULL</ReqID>
      | <InitValue>0</InitValue>
  CarCfg_CarCfg376 : Inport  Description=|  Port=342  OutDataTypeStr=Nr2  PortDimensions=1
      | ISMOBILITY
      | <to>All</to>
      | <typ>Internal</typ>
      | <ReqID>NULL</ReqID>
      | <InitValue>0</InitValue>
  CarCfg_CarCfg38 : Inport  Description=|  Port=343  OutDataTypeStr=Nr2  PortDimensions=1
      | ENGINE COOLING FAN PERFORMANCE
      | <to>All</to>
      | <typ>Internal</typ>
      | <ReqID>NULL</ReqID>
      | <InitValue>0</InitValue>
  CarCfg_CarCfg39 : Inport  Description=|  Port=344  OutDataTypeStr=Nr2  PortDimensions=1
      | SHIFT BY WIRE
      | <to>All</to>
      | <typ>Internal</typ>
      | <ReqID>NULL</ReqID>
      | <InitValue>0</InitValue>
  CarCfg_CarCfg4 : Inport  Description=|  Port=345  OutDataTypeStr=Nr2  PortDimensions=1
      | ELECTRIC DRIVELINE
      | <to>All</to>
      | <typ>Internal</typ>
      | <ReqID>NULL</ReqID>
      | <InitValue>0</InitValue>
  CarCfg_CarCfg401 : Inport  Description=|  Port=346  OutDataTypeStr=Nr2  PortDimensions=1
      | RAIN SENSOR FUNCTION
      | <to>All</to>
      | <typ>Internal</typ>
      | <ReqID>NULL</ReqID>
      | <InitValue>0</InitValue>
  CarCfg_CarCfg407 : Inport  Description=|  Port=347  OutDataTypeStr=Nr2  PortDimensions=1
      | COOLANT LEVEL INDICATION
      | <to>All</to>
      | <typ>Internal</typ>
      | <ReqID>NULL</ReqID>
      | <InitValue>0</InitValue>
  CarCfg_CarCfg408 : Inport  Description=|  Port=348  OutDataTypeStr=Nr2  PortDimensions=1
      | REAR SUSPENSION TYPE
      | <to>All</to>
      | <typ>Internal</typ>
      | <ReqID>NULL</ReqID>
      | <InitValue>0</InitValue>
  CarCfg_CarCfg409 : Inport  Description=|  Port=349  OutDataTypeStr=Nr2  PortDimensions=1
      | IMU SENSOR CONFIGURATION
      | <to>All</to>
      | <typ>Internal</typ>
      | <ReqID>NULL</ReqID>
      | <InitValue>0</InitValue>
  CarCfg_CarCfg41 : Inport  Description=|  Port=350  OutDataTypeStr=Nr2  PortDimensions=1
      | GEAR SHIFT PADDLES
      | <to>All</to>
      | <typ>Internal</typ>
      | <ReqID>NULL</ReqID>
      | <InitValue>0</InitValue>
  CarCfg_CarCfg410 : Inport  Description=|  Port=351  OutDataTypeStr=Nr2  PortDimensions=1
      | VACUUM SENSOR
      | <to>All</to>
      | <typ>Internal</typ>
      | <ReqID>NULL</ReqID>
      | <InitValue>0</InitValue>
  CarCfg_CarCfg413 : Inport  Description=|  Port=352  OutDataTypeStr=Nr2  PortDimensions=1
      | CONVENIENCE START
      | <to>All</to>
      | <typ>Internal</typ>
      | <ReqID>NULL</ReqID>
      | <InitValue>0</InitValue>
  CarCfg_CarCfg414 : Inport  Description=|  Port=353  OutDataTypeStr=Nr2  PortDimensions=1
      | CONVENIENCE STOP
      | <to>All</to>
      | <typ>Internal</typ>
      | <ReqID>NULL</ReqID>
      | <InitValue>0</InitValue>
  CarCfg_CarCfg415 : Inport  Description=|  Port=354  OutDataTypeStr=Nr2  PortDimensions=1
      | OCCUPANT DETECTION SENSOR DRIVER SEAT
      | <to>All</to>
      | <typ>Internal</typ>
      | <ReqID>NULL</ReqID>
      | <InitValue>0</InitValue>
  CarCfg_CarCfg42 : Inport  Description=|  Port=355  OutDataTypeStr=Nr2  PortDimensions=1
      | PART NUMBER FORMAT
      | <to>All</to>
      | <typ>Internal</typ>
      | <ReqID>NULL</ReqID>
      | <InitValue>0</InitValue>
  CarCfg_CarCfg43 : Inport  Description=|  Port=356  OutDataTypeStr=Nr2  PortDimensions=1
      | MAX VEHICLE SPEED LIMITATION
      | <to>All</to>
      | <typ>Internal</typ>
      | <ReqID>NULL</ReqID>
      | <InitValue>0</InitValue>
  CarCfg_CarCfg44 : Inport  Description=|  Port=357  OutDataTypeStr=Nr2  PortDimensions=1
      | STOP IN SPEED VELOCITY
      | <to>All</to>
      | <typ>Internal</typ>
      | <ReqID>NULL</ReqID>
      | <InitValue>0</InitValue>
  CarCfg_CarCfg441 : Inport  Description=|  Port=358  OutDataTypeStr=Nr2  PortDimensions=1
      | VEHICLE TYPE
      | <to>All</to>
      | <typ>Internal</typ>
      | <ReqID>NULL</ReqID>
      | <InitValue>0</InitValue>
  CarCfg_CarCfg449 : Inport  Description=|  Port=359  OutDataTypeStr=Nr2  PortDimensions=1
      | CSD VARIANTS
      | <to>All</to>
      | <typ>Internal</typ>
      | <ReqID>NULL</ReqID>
      | <InitValue>0</InitValue>
  CarCfg_CarCfg45 : Inport  Description=|  Port=360  OutDataTypeStr=Nr2  PortDimensions=1
      | REMOTE ENGINE START PRECOND
      | <to>All</to>
      | <typ>Internal</typ>
      | <ReqID>NULL</ReqID>
      | <InitValue>0</InitValue>
  CarCfg_CarCfg450 : Inport  Description=|  Port=361  OutDataTypeStr=Nr2  PortDimensions=1
      | WIRELESS PHONE CHARGING (WPC) AND NEAR FIELD COMMUNICATION (NFC)
      | <to>All</to>
      | <typ>Internal</typ>
      | <ReqID>NULL</ReqID>
      | <InitValue>0</InitValue>
  CarCfg_CarCfg451 : Inport  Description=|  Port=362  OutDataTypeStr=Nr2  PortDimensions=1
      | AUDIO & AMP LEVEL
      | <to>All</to>
      | <typ>Internal</typ>
      | <ReqID>NULL</ReqID>
      | <InitValue>0</InitValue>
  CarCfg_CarCfg452 : Inport  Description=|  Port=363  OutDataTypeStr=Nr2  PortDimensions=1
      | DRIVE MODE SWITCH
      | <to>All</to>
      | <typ>Internal</typ>
      | <ReqID>NULL</ReqID>
      | <InitValue>0</InitValue>
  CarCfg_CarCfg453 : Inport  Description=|  Port=364  OutDataTypeStr=Nr2  PortDimensions=1
      | GLOBAL NAVIGATION SATELLITE SYSTEM
      | <to>All</to>
      | <typ>Internal</typ>
      | <ReqID>NULL</ReqID>
      | <InitValue>0</InitValue>
  CarCfg_CarCfg454 : Inport  Description=|  Port=365  OutDataTypeStr=Nr2  PortDimensions=1
      | AM RADIO FUNCTION
      | <to>All</to>
      | <typ>Internal</typ>
      | <ReqID>NULL</ReqID>
      | <InitValue>0</InitValue>
  CarCfg_CarCfg455 : Inport  Description=|  Port=366  OutDataTypeStr=Nr2  PortDimensions=1
      | MIRACAST AND MIRRORLINK FUNCTION
      | <to>All</to>
      | <typ>Internal</typ>
      | <ReqID>NULL</ReqID>
      | <InitValue>0</InitValue>
  CarCfg_CarCfg456 : Inport  Description=|  Port=367  OutDataTypeStr=Nr2  PortDimensions=1
      | BELT PRE-TENSIONER 2ND ROW
      | <to>All</to>
      | <typ>Internal</typ>
      | <ReqID>NULL</ReqID>
      | <InitValue>0</InitValue>
  CarCfg_CarCfg457 : Inport  Description=|  Port=368  OutDataTypeStr=Nr2  PortDimensions=1
      | INFLATABLE CURTAINS
      | <to>All</to>
      | <typ>Internal</typ>
      | <ReqID>NULL</ReqID>
      | <InitValue>0</InitValue>
  CarCfg_CarCfg458 : Inport  Description=|  Port=369  OutDataTypeStr=Nr2  PortDimensions=1
      | FRONT SIDE AIRBAGS
      | <to>All</to>
      | <typ>Internal</typ>
      | <ReqID>NULL</ReqID>
      | <InitValue>0</InitValue>
  CarCfg_CarCfg459 : Inport  Description=|  Port=370  OutDataTypeStr=Nr2  PortDimensions=1
      | STEERING WHEEL BUTTONS
      | <to>All</to>
      | <typ>Internal</typ>
      | <ReqID>NULL</ReqID>
      | <InitValue>0</InitValue>
  CarCfg_CarCfg46 : Inport  Description=|  Port=371  OutDataTypeStr=Nr2  PortDimensions=1
      | DIAGNOSIS SYSTEM
      | <to>All</to>
      | <typ>Internal</typ>
      | <ReqID>NULL</ReqID>
      | <InitValue>0</InitValue>
  CarCfg_CarCfg460 : Inport  Description=|  Port=372  OutDataTypeStr=Nr2  PortDimensions=1
      | AUDIO SPEAKERS
      | <to>All</to>
      | <typ>Internal</typ>
      | <ReqID>NULL</ReqID>
      | <InitValue>0</InitValue>
  CarCfg_CarCfg461 : Inport  Description=|  Port=373  OutDataTypeStr=Nr2  PortDimensions=1
      | APPROVED CELLULAR STANDARDS
      | <to>All</to>
      | <typ>Internal</typ>
      | <ReqID>NULL</ReqID>
      | <InitValue>0</InitValue>
  CarCfg_CarCfg462 : Inport  Description=|  Port=374  OutDataTypeStr=Nr2  PortDimensions=1
      | HEATING MAT VARIANTS
      | <to>All</to>
      | <typ>Internal</typ>
      | <ReqID>NULL</ReqID>
      | <InitValue>0</InitValue>
  CarCfg_CarCfg463 : Inport  Description=|  Port=375  OutDataTypeStr=Nr2  PortDimensions=1
      | MEMORY IN REAR VIEW MIRRORS
      | <to>All</to>
      | <typ>Internal</typ>
      | <ReqID>NULL</ReqID>
      | <InitValue>0</InitValue>
  CarCfg_CarCfg464 : Inport  Description=|  Port=376  OutDataTypeStr=Nr2  PortDimensions=1
      | SUPERCAPACITOR
      | <to>All</to>
      | <typ>Internal</typ>
      | <ReqID>NULL</ReqID>
      | <InitValue>0</InitValue>
  CarCfg_CarCfg465 : Inport  Description=|  Port=377  OutDataTypeStr=Nr2  PortDimensions=1
      | AIRBORNE PARTICULATE MATTER APMA
      | <to>All</to>
      | <typ>Internal</typ>
      | <ReqID>NULL</ReqID>
      | <InitValue>0</InitValue>
  CarCfg_CarCfg466 : Inport  Description=|  Port=378  OutDataTypeStr=Nr2  PortDimensions=1
      | AMPLIFIER VARIANT
      | <to>All</to>
      | <typ>Internal</typ>
      | <ReqID>NULL</ReqID>
      | <InitValue>0</InitValue>
  CarCfg_CarCfg467 : Inport  Description=|  Port=379  OutDataTypeStr=Nr2  PortDimensions=1
      | SHUTFACE BUTTONS ON TAILGATE/BOOTLID
      | <to>All</to>
      | <typ>Internal</typ>
      | <ReqID>NULL</ReqID>
      | <InitValue>0</InitValue>
  CarCfg_CarCfg468 : Inport  Description=|  Port=380  OutDataTypeStr=Nr2  PortDimensions=1
      | FIRST ROW TILT MOTORS
      | <to>All</to>
      | <typ>Internal</typ>
      | <ReqID>NULL</ReqID>
      | <InitValue>0</InitValue>
  CarCfg_CarCfg469 : Inport  Description=|  Port=381  OutDataTypeStr=Nr2  PortDimensions=1